simulink model "MX_Gateway"  (Simulink 9.0)
settings: Solver=FixedStepDiscrete  StartTime=0.0  StopTime=100  FixedStep=0.01  RelTol=1e-3
values from neighbouring files (symbols this model uses):
  ACTIVE = Bobcat.Parameter; CreateDefine( ACTIVE                         ,1                          ,'Define' ,'defines.h' )    [defines.m]
  ACTIVE_MODE_ONE = Bobcat.Parameter; CreateDefine( ACTIVE_MODE_ONE                ,19                         ,'Define' ,'defines.h' )    [defines.m]
  AUTHENTICATED = Bobcat.Parameter; CreateDefine( AUTHENTICATED                  ,1                          ,'Define' ,'defines.h' )    [defines.m]
  AUX_EMEA_CONTROLS = Bobcat.Parameter; CreateDefine( AUX_EMEA_CONTROLS  ,1   ,'Define' ,'defines.h' )    [enums.m]
  AUX_FLOW_HIGH = Bobcat.Parameter; CreateDefine( AUX_FLOW_HIGH           ,4  ,'Define' ,'defines.h' )    [enums.m]
  AUX_FLOW_INACTIVE = Bobcat.Parameter; CreateDefine( AUX_FLOW_INACTIVE       ,0  ,'Define' ,'defines.h' )    [enums.m]
  AUX_FLOW_LOW = Bobcat.Parameter; CreateDefine( AUX_FLOW_LOW            ,1  ,'Define' ,'defines.h' )    [enums.m]
  AUX_FLOW_MEDIUM = Bobcat.Parameter; CreateDefine( AUX_FLOW_MEDIUM         ,2  ,'Define' ,'defines.h' )    [enums.m]
  AUX_FLOW_STANDARD = Bobcat.Parameter; CreateDefine( AUX_FLOW_STANDARD       ,3  ,'Define' ,'defines.h' )    [enums.m]
  AUX_NON_FUNCTIONAL = Bobcat.Parameter; CreateDefine( AUX_NON_FUNCTIONAL ,255 ,'Define' ,'defines.h' )    [enums.m]
  BASE_ACTIVE = Bobcat.Parameter; CreateDefine( BASE_ACTIVE                    ,253                        ,'Define' ,'defines.h' )    [defines.m]
  BASE_DETENT = Bobcat.Parameter; CreateDefine( BASE_DETENT                    ,1                          ,'Define' ,'defines.h' )    [defines.m]
  BEEP_NONE = Bobcat.Parameter; CreateDefine( BEEP_NONE                      ,255                        ,'Define' ,'defines.h' )    [defines.m]
  CD_AIR_FILTER_DEBOUNCE_NORMAL = Bobcat.Parameter; CreateAliasCD( CD_AIR_FILTER_DEBOUNCE_NORMAL                        ,uint16(500)                ,'ConstantDataPointer' ,'CONSTANT_DATA' ,'AIR_FILTER_DEBOUNCE_NORMAL')    [cdata.m]    (also in hc12_cdata.m; m4_cdata.m)
  CD_AIR_FILTER_DEBOUNCE_NOT_CONNECTED = Bobcat.Parameter; CreateAliasCD( CD_AIR_FILTER_DEBOUNCE_NOT_CONNECTED                 ,uint16(3000)               ,'ConstantDataPointer' ,'CONSTANT_DATA' ,'AIR_FILTER_DEBOUNCE_NOT_CONNECTED')    [cdata.m]    (also in hc12_cdata.m; m4_cdata.m)
  CD_AIR_FILTER_DEBOUNCE_PLUGGED = Bobcat.Parameter; CreateAliasCD( CD_AIR_FILTER_DEBOUNCE_PLUGGED                       ,uint16(3000)               ,'ConstantDataPointer' ,'CONSTANT_DATA' ,'AIR_FILTER_DEBOUNCE_PLUGGED')    [cdata.m]    (also in hc12_cdata.m; m4_cdata.m)
  CD_AIR_FILTER_FUNCTION = Bobcat.Parameter; CreateAliasCD( CD_AIR_FILTER_FUNCTION                               ,uint8(255)                 ,'ConstantDataPointer' ,'CONSTANT_DATA' ,'AIR_FILTER_FUNCTION')    [cdata.m]    (also in hc12_cdata.m; m4_cdata.m)
  CD_AIR_FILTER_PLUGGED_WEIGHT = Bobcat.Parameter; CreateAliasCD( CD_AIR_FILTER_PLUGGED_WEIGHT                         ,uint8(2)                   ,'ConstantDataPointer' ,'CONSTANT_DATA' ,'AIR_FILTER_PLUGGED_WEIGHT')    [cdata.m]    (also in hc12_cdata.m; m4_cdata.m)
  CD_AIR_FILTER_UNPLUGGED_WEIGHT = Bobcat.Parameter; CreateAliasCD( CD_AIR_FILTER_UNPLUGGED_WEIGHT                       ,uint8(1)                   ,'ConstantDataPointer' ,'CONSTANT_DATA' ,'AIR_FILTER_UNPLUGGED_WEIGHT')    [cdata.m]    (also in hc12_cdata.m; m4_cdata.m)
  CD_AUTOIDLE_ARMBAR_DELAY = Bobcat.Parameter; CreateAliasCD( CD_AUTOIDLE_ARMBAR_DELAY                             ,uint8(2)                   ,'ConstantDataPointer' ,'CONSTANT_DATA' ,'AUTOIDLE_ARMBAR_DELAY')    [cdata.m]    (also in hc12_cdata.m; m4_cdata.m)
  CD_AUTOIDLE_FUNCTION = Bobcat.Parameter; CreateAliasCD( CD_AUTOIDLE_FUNCTION                                 ,uint8(255)                 ,'ConstantDataPointer' ,'CONSTANT_DATA' ,'AUTOIDLE_FUNCTION')    [cdata.m]    (also in hc12_cdata.m; m4_cdata.m)
  CD_AUTOIDLE_IDLE_DELAY = Bobcat.Parameter; CreateAliasCD( CD_AUTOIDLE_IDLE_DELAY                               ,uint8(40)                  ,'ConstantDataPointer' ,'CONSTANT_DATA' ,'AUTOIDLE_IDLE_DELAY')    [cdata.m]    (also in hc12_cdata.m; m4_cdata.m)
  CD_AUTOIDLE_LOW_IDLE_START = Bobcat.Parameter; CreateAliasCD( CD_AUTOIDLE_LOW_IDLE_START                           ,uint8(255)                 ,'ConstantDataPointer' ,'CONSTANT_DATA' ,'AUTOIDLE_LOW_IDLE_START')    [cdata.m]    (also in hc12_cdata.m; m4_cdata.m)
  CD_AUTOIDLE_PRESSURE_OFF = Bobcat.Parameter; CreateAliasCD( CD_AUTOIDLE_PRESSURE_OFF                             ,uint16(300)                ,'ConstantDataPointer' ,'CONSTANT_DATA' ,'AUTOIDLE_PRESSURE_OFF')    [cdata.m]    (also in hc12_cdata.m; m4_cdata.m)
  CD_AUTOIDLE_PRESSURE_ON = Bobcat.Parameter; CreateAliasCD( CD_AUTOIDLE_PRESSURE_ON                              ,uint16(270)                ,'ConstantDataPointer' ,'CONSTANT_DATA' ,'AUTOIDLE_PRESSURE_ON')    [cdata.m]    (also in hc12_cdata.m; m4_cdata.m)
  CD_AUTOSHIFT_FUNCTION = Bobcat.Parameter; CreateAliasCD( CD_AUTOSHIFT_FUNCTION                                ,uint8(255)                 ,'ConstantDataPointer' ,'CONSTANT_DATA' ,'AUTOSHIFT_FUNCTION')    [cdata.m]    (also in hc12_cdata.m; m4_cdata.m)
  CD_AUTOSHIFT_PHIGH1 = Bobcat.Parameter; CreateAliasCD( CD_AUTOSHIFT_PHIGH1                                  ,uint16(205)                ,'ConstantDataPointer' ,'CONSTANT_DATA' ,'AUTOSHIFT_PHIGH1')    [cdata.m]    (also in hc12_cdata.m; m4_cdata.m)
  CD_AUTOSHIFT_PHIGH2 = Bobcat.Parameter; CreateAliasCD( CD_AUTOSHIFT_PHIGH2                                  ,uint16(193)                ,'ConstantDataPointer' ,'CONSTANT_DATA' ,'AUTOSHIFT_PHIGH2')    [cdata.m]    (also in hc12_cdata.m; m4_cdata.m)
  CD_AUTOSHIFT_PHIGH_TEMP1 = Bobcat.Parameter; CreateAliasCD( CD_AUTOSHIFT_PHIGH_TEMP1                             ,int16(70)                  ,'ConstantDataPointer' ,'CONSTANT_DATA' ,'AUTOSHIFT_PHIGH_TEMP1')    [cdata.m]    (also in hc12_cdata.m; m4_cdata.m)
  CD_AUTOSHIFT_PHIGH_TEMP2 = Bobcat.Parameter; CreateAliasCD( CD_AUTOSHIFT_PHIGH_TEMP2                             ,int16(160)                 ,'ConstantDataPointer' ,'CONSTANT_DATA' ,'AUTOSHIFT_PHIGH_TEMP2')    [cdata.m]    (also in hc12_cdata.m; m4_cdata.m)
  CD_AUTOSHIFT_PLOW1 = Bobcat.Parameter; CreateAliasCD( CD_AUTOSHIFT_PLOW1                                   ,uint16(130)                ,'ConstantDataPointer' ,'CONSTANT_DATA' ,'AUTOSHIFT_PLOW1')    [cdata.m]    (also in hc12_cdata.m; m4_cdata.m)
  CD_AUTOSHIFT_PLOW2 = Bobcat.Parameter; CreateAliasCD( CD_AUTOSHIFT_PLOW2                                   ,uint16(100)                ,'ConstantDataPointer' ,'CONSTANT_DATA' ,'AUTOSHIFT_PLOW2')    [cdata.m]    (also in hc12_cdata.m; m4_cdata.m)
  CD_AUTOSHIFT_PLOW_TEMP1 = Bobcat.Parameter; CreateAliasCD( CD_AUTOSHIFT_PLOW_TEMP1                              ,int16(20)                  ,'ConstantDataPointer' ,'CONSTANT_DATA' ,'AUTOSHIFT_PLOW_TEMP1')    [cdata.m]    (also in hc12_cdata.m; m4_cdata.m)
  CD_AUTOSHIFT_PLOW_TEMP2 = Bobcat.Parameter; CreateAliasCD( CD_AUTOSHIFT_PLOW_TEMP2                              ,int16(80)                  ,'ConstantDataPointer' ,'CONSTANT_DATA' ,'AUTOSHIFT_PLOW_TEMP2')    [cdata.m]    (also in hc12_cdata.m; m4_cdata.m)
  CD_AUTOSHIFT_TRAVEL_DELAY = Bobcat.Parameter; CreateAliasCD( CD_AUTOSHIFT_TRAVEL_DELAY                            ,uint8(32)                  ,'ConstantDataPointer' ,'CONSTANT_DATA' ,'AUTOSHIFT_TRAVEL_DELAY')    [cdata.m]    (also in hc12_cdata.m; m4_cdata.m)
  CD_AUX2_CRACKPOINT_CURRENT = Bobcat.Parameter; CreateAliasCD( CD_AUX2_CRACKPOINT_CURRENT                           ,fi(0,0,16,12,'hex','0c28') ,'ConstantDataPointer' ,'CONSTANT_DATA' ,'AUX2_CRACKPOINT_CURRENT')    [cdata.m]    (also in hc12_cdata.m; m4_cdata.m)
  CD_AUX2_FLOW_LOW_FUNCTION = Bobcat.Parameter; CreateAliasCD( CD_AUX2_FLOW_LOW_FUNCTION                            ,uint8(255)                 ,'ConstantDataPointer' ,'CONSTANT_DATA' ,'AUX2_FLOW_LOW_FUNCTION')    [cdata.m]    (also in hc12_cdata.m; m4_cdata.m)
  CD_AUX2_FLOW_MED_FUNCTION = Bobcat.Parameter; CreateAliasCD( CD_AUX2_FLOW_MED_FUNCTION                            ,uint8(255)                 ,'ConstantDataPointer' ,'CONSTANT_DATA' ,'AUX2_FLOW_MED_FUNCTION')    [cdata.m]    (also in hc12_cdata.m; m4_cdata.m)
  CD_AUX2_HI_FLOW_PERCENT = Bobcat.Parameter; CreateAliasCD( CD_AUX2_HI_FLOW_PERCENT                              ,fi(0,0,16,16,'hex','ffff') ,'ConstantDataPointer' ,'CONSTANT_DATA' ,'AUX2_HI_FLOW_PERCENT')    [cdata.m]    (also in hc12_cdata.m; m4_cdata.m)
  CD_AUX2_LOW_FLOW_PERCENT = Bobcat.Parameter; CreateAliasCD( CD_AUX2_LOW_FLOW_PERCENT                             ,fi(0,0,16,16,'hex','7333') ,'ConstantDataPointer' ,'CONSTANT_DATA' ,'AUX2_LOW_FLOW_PERCENT')    [cdata.m]    (also in hc12_cdata.m; m4_cdata.m)
  CD_AUX2_MAX_FLOW_CURRENT = Bobcat.Parameter; CreateAliasCD( CD_AUX2_MAX_FLOW_CURRENT                             ,fi(0,0,16,12,'hex','14cc') ,'ConstantDataPointer' ,'CONSTANT_DATA' ,'AUX2_MAX_FLOW_CURRENT')    [cdata.m]    (also in hc12_cdata.m; m4_cdata.m)
  CD_AUX2_MED_FLOW_PERCENT = Bobcat.Parameter; CreateAliasCD( CD_AUX2_MED_FLOW_PERCENT                             ,fi(0,0,16,16,'hex','9999') ,'ConstantDataPointer' ,'CONSTANT_DATA' ,'AUX2_MED_FLOW_PERCENT')    [cdata.m]    (also in hc12_cdata.m; m4_cdata.m)
  CD_AUX_CRACKPOINT_CURRENT = Bobcat.Parameter; CreateAliasCD( CD_AUX_CRACKPOINT_CURRENT                            ,fi(0,0,16,12,'hex','0000') ,'ConstantDataPointer' ,'CONSTANT_DATA' ,'AUX_CRACKPOINT_CURRENT')    [cdata.m]    (also in hc12_cdata.m; m4_cdata.m)
  CD_AUX_DETENT_FLASH_TIME = Bobcat.Parameter; CreateAliasCD( CD_AUX_DETENT_FLASH_TIME                             ,uint16(72)                 ,'ConstantDataPointer' ,'CONSTANT_DATA' ,'AUX_DETENT_FLASH_TIME')    [cdata.m]    (also in hc12_cdata.m; m4_cdata.m)
  CD_AUX_DETENT_HOLD_TIME = Bobcat.Parameter; CreateAliasCD( CD_AUX_DETENT_HOLD_TIME                              ,uint16(100)                ,'ConstantDataPointer' ,'CONSTANT_DATA' ,'AUX_DETENT_HOLD_TIME')    [cdata.m]    (also in hc12_cdata.m; m4_cdata.m)
  CD_AUX_FULL_ON_AUX1 = Bobcat.Parameter; CreateAliasCD( CD_AUX_FULL_ON_AUX1                                  ,fi(0,0,16,12,'hex','10cd') ,'ConstantDataPointer' ,'CONSTANT_DATA' ,'AUX_FULL_ON_AUX1')    [cdata.m]    (also in hc12_cdata.m; m4_cdata.m)
  CD_AUX_FULL_ON_AUX2 = Bobcat.Parameter; CreateAliasCD( CD_AUX_FULL_ON_AUX2                                  ,fi(0,0,16,12,'hex','139d') ,'ConstantDataPointer' ,'CONSTANT_DATA' ,'AUX_FULL_ON_AUX2')    [cdata.m]    (also in hc12_cdata.m; m4_cdata.m)
  CD_AUX_FULL_ON_AUX3 = Bobcat.Parameter; CreateAliasCD( CD_AUX_FULL_ON_AUX3                                  ,fi(0,0,16,12,'hex','17d7') ,'ConstantDataPointer' ,'CONSTANT_DATA' ,'AUX_FULL_ON_AUX3')    [cdata.m]    (also in hc12_cdata.m; m4_cdata.m)
  CD_AUX_FUNCTION = Bobcat.Parameter; CreateAliasCD( CD_AUX_FUNCTION                                      ,uint8(0)                   ,'ConstantDataPointer' ,'CONSTANT_DATA' ,'AUX_FUNCTION')    [cdata.m]    (also in hc12_cdata.m; m4_cdata.m)
  CD_AUX_RATE_LIMIT_CRACKPOINT = Bobcat.Parameter; CreateAliasCD( CD_AUX_RATE_LIMIT_CRACKPOINT                         ,fi(0,0,16,12,'hex','0800') ,'ConstantDataPointer' ,'CONSTANT_DATA' ,'AUX_RATE_LIMIT_CRACKPOINT')    [cdata.m]    (also in hc12_cdata.m; m4_cdata.m)
  CD_AUX_RATE_LIMIT_CRACKPOINT_RATE = Bobcat.Parameter; CreateAliasCD( CD_AUX_RATE_LIMIT_CRACKPOINT_RATE                    ,fi(0,1,32,12,'int',102400) ,'ConstantDataPointer' ,'CONSTANT_DATA' ,'AUX_RATE_LIMIT_CRACKPOINT_RATE')    [cdata.m]    (also in hc12_cdata.m; m4_cdata.m)
  CD_AUX_RATE_LIMIT_FUNCTION = Bobcat.Parameter; CreateAliasCD( CD_AUX_RATE_LIMIT_FUNCTION                           ,uint8(255)                 ,'ConstantDataPointer' ,'CONSTANT_DATA' ,'AUX_RATE_LIMIT_FUNCTION')    [cdata.m]    (also in hc12_cdata.m; m4_cdata.m)
  CD_AUX_RATE_LIMIT_MAX_ENG_SPD = Bobcat.Parameter; CreateAliasCD( CD_AUX_RATE_LIMIT_MAX_ENG_SPD                        ,uint16(1600)               ,'ConstantDataPointer' ,'CONSTANT_DATA' ,'AUX_RATE_LIMIT_MAX_ENG_SPD')    [cdata.m]    (also in hc12_cdata.m; m4_cdata.m)
  CD_AUX_RATE_LIMIT_MIN_ENG_SPD = Bobcat.Parameter; CreateAliasCD( CD_AUX_RATE_LIMIT_MIN_ENG_SPD                        ,uint16(100)                ,'ConstantDataPointer' ,'CONSTANT_DATA' ,'AUX_RATE_LIMIT_MIN_ENG_SPD')    [cdata.m]    (also in hc12_cdata.m; m4_cdata.m)
  CD_AUX_RATE_LIMIT_NORMAL_RATE = Bobcat.Parameter; CreateAliasCD( CD_AUX_RATE_LIMIT_NORMAL_RATE                        ,fi(0,1,32,12,'int',2048)   ,'ConstantDataPointer' ,'CONSTANT_DATA' ,'AUX_RATE_LIMIT_NORMAL_RATE')    [cdata.m]    (also in hc12_cdata.m; m4_cdata.m)
  CD_AUXPOT_CAL_DEADBAND_HIGH = Bobcat.Parameter; CreateAliasCD( CD_AUXPOT_CAL_DEADBAND_HIGH                          ,uint16(574)                ,'ConstantDataPointer' ,'CONSTANT_DATA' ,'AUXPOT_CAL_DEADBAND_HIGH')    [cdata.m]    (also in hc12_cdata.m; m4_cdata.m)
  CD_AUXPOT_CAL_DEADBAND_LOW = Bobcat.Parameter; CreateAliasCD( CD_AUXPOT_CAL_DEADBAND_LOW                           ,uint16(424)                ,'ConstantDataPointer' ,'CONSTANT_DATA' ,'AUXPOT_CAL_DEADBAND_LOW')    [cdata.m]    (also in hc12_cdata.m; m4_cdata.m)
  CD_AUXPOT_CENTER_RATIO = Bobcat.Parameter; CreateAliasCD( CD_AUXPOT_CENTER_RATIO                               ,uint16(500)                ,'ConstantDataPointer' ,'CONSTANT_DATA' ,'AUXPOT_CENTER_RATIO')    [cdata.m]    (also in hc12_cdata.m; m4_cdata.m)
  CD_AUXPOT_DEADBAND = Bobcat.Parameter; CreateAliasCD( CD_AUXPOT_DEADBAND                                   ,uint8(38)                  ,'ConstantDataPointer' ,'CONSTANT_DATA' ,'AUXPOT_DEADBAND')    [cdata.m]    (also in hc12_cdata.m; m4_cdata.m)
  CD_AUXPOT_FUNCTION = Bobcat.Parameter; CreateAliasCD( CD_AUXPOT_FUNCTION                                   ,uint8(0)                   ,'ConstantDataPointer' ,'CONSTANT_DATA' ,'AUXPOT_FUNCTION')    [cdata.m]    (also in hc12_cdata.m; m4_cdata.m)
  CD_AUXPOT_RATIO_GAIN = Bobcat.Parameter; CreateAliasCD( CD_AUXPOT_RATIO_GAIN                                 ,uint16(1000)               ,'ConstantDataPointer' ,'CONSTANT_DATA' ,'AUXPOT_RATIO_GAIN')    [cdata.m]    (also in hc12_cdata.m; m4_cdata.m)
  CD_CAN_THROTTLE_FUNCTION = Bobcat.Parameter; CreateAliasCD( CD_CAN_THROTTLE_FUNCTION                             ,uint8(255)                 ,'ConstantDataPointer' ,'CONSTANT_DATA' ,'CAN_THROTTLE_FUNCTION')    [cdata.m]    (also in hc12_cdata.m; m4_cdata.m)
  CD_CAN_THROTTLE_REVERSE = Bobcat.Parameter; CreateAliasCD( CD_CAN_THROTTLE_REVERSE                              ,uint8(255)                 ,'ConstantDataPointer' ,'CONSTANT_DATA' ,'CAN_THROTTLE_REVERSE')    [cdata.m]    (also in hc12_cdata.m; m4_cdata.m)
  CD_CONSOLE_DEBOUNCE_ABSENT = Bobcat.Parameter; CreateAliasCD( CD_CONSOLE_DEBOUNCE_ABSENT                           ,uint16(12)                 ,'ConstantDataPointer' ,'CONSTANT_DATA' ,'CONSOLE_DEBOUNCE_ABSENT')    [cdata.m]    (also in hc12_cdata.m; m4_cdata.m)
  CD_CONSOLE_DEBOUNCE_OORH = Bobcat.Parameter; CreateAliasCD( CD_CONSOLE_DEBOUNCE_OORH                             ,uint16(12)                 ,'ConstantDataPointer' ,'CONSTANT_DATA' ,'CONSOLE_DEBOUNCE_OORH')    [cdata.m]    (also in hc12_cdata.m; m4_cdata.m)
  CD_CONSOLE_DEBOUNCE_OORL = Bobcat.Parameter; CreateAliasCD( CD_CONSOLE_DEBOUNCE_OORL                             ,uint16(12)                 ,'ConstantDataPointer' ,'CONSTANT_DATA' ,'CONSOLE_DEBOUNCE_OORL')    [cdata.m]    (also in hc12_cdata.m; m4_cdata.m)
  CD_CONSOLE_DEBOUNCE_PRESENT = Bobcat.Parameter; CreateAliasCD( CD_CONSOLE_DEBOUNCE_PRESENT                          ,uint16(12)                 ,'ConstantDataPointer' ,'CONSTANT_DATA' ,'CONSOLE_DEBOUNCE_PRESENT')    [cdata.m]    (also in hc12_cdata.m; m4_cdata.m)
  CD_CONSOLE_FUNCTION = Bobcat.Parameter; CreateAliasCD( CD_CONSOLE_FUNCTION                                  ,uint8(0)                   ,'ConstantDataPointer' ,'CONSTANT_DATA' ,'CONSOLE_FUNCTION')    [cdata.m]    (also in hc12_cdata.m; m4_cdata.m)
  CD_CONSOLE_OORH = Bobcat.Parameter; CreateAliasCD( CD_CONSOLE_OORH                                      ,uint16(740)                ,'ConstantDataPointer' ,'CONSTANT_DATA' ,'CONSOLE_OORH')    [cdata.m]    (also in hc12_cdata.m; m4_cdata.m)
  CD_CONSOLE_OORL = Bobcat.Parameter; CreateAliasCD( CD_CONSOLE_OORL                                      ,uint16(10)                 ,'ConstantDataPointer' ,'CONSTANT_DATA' ,'CONSOLE_OORL')    [cdata.m]    (also in hc12_cdata.m; m4_cdata.m)
  CD_CONSOLE_PRESENT_HIGH = Bobcat.Parameter; CreateAliasCD( CD_CONSOLE_PRESENT_HIGH                              ,uint16(515)                ,'ConstantDataPointer' ,'CONSTANT_DATA' ,'CONSOLE_PRESENT_HIGH')    [cdata.m]    (also in hc12_cdata.m; m4_cdata.m)
  CD_CONSOLE_PRESENT_LOW = Bobcat.Parameter; CreateAliasCD( CD_CONSOLE_PRESENT_LOW                               ,uint16(375)                ,'ConstantDataPointer' ,'CONSTANT_DATA' ,'CONSOLE_PRESENT_LOW')    [cdata.m]    (also in hc12_cdata.m; m4_cdata.m)
  CD_DELUXE_GEN5_5_FUNCTION = Bobcat.Parameter; CreateAliasCD( CD_DELUXE_GEN5_5_FUNCTION                            ,uint8(255)                 ,'ConstantDataPointer' ,'CONSTANT_DATA' ,'DELUXE_GEN5_5_FUNCTION')    [cdata.m]    (also in hc12_cdata.m; m4_cdata.m)
  CD_DELUXE_GEN5_FUNCTION = Bobcat.Parameter; CreateAliasCD( CD_DELUXE_GEN5_FUNCTION                              ,uint8(255)                 ,'ConstantDataPointer' ,'CONSTANT_DATA' ,'DELUXE_GEN5_FUNCTION')    [cdata.m]    (also in hc12_cdata.m; m4_cdata.m)
  CD_DERATE_ENGINE_SPEED_DEBOUNCE_HIGH = Bobcat.Parameter; CreateAliasCD( CD_DERATE_ENGINE_SPEED_DEBOUNCE_HIGH                 ,uint16(200)                ,'ConstantDataPointer' ,'CONSTANT_DATA' ,'DERATE_ENGINE_SPEED_DEBOUNCE_HIGH')    [cdata.m]    (also in hc12_cdata.m; m4_cdata.m)
  CD_DERATE_ENGINE_SPEED_DEBOUNCE_SHUTDOWN = Bobcat.Parameter; CreateAliasCD( CD_DERATE_ENGINE_SPEED_DEBOUNCE_SHUTDOWN             ,uint16(1000)               ,'ConstantDataPointer' ,'CONSTANT_DATA' ,'DERATE_ENGINE_SPEED_DEBOUNCE_SHUTDOWN')    [cdata.m]    (also in hc12_cdata.m; m4_cdata.m)
  CD_DERATE_ENGINE_SPEED_HIGH_OFFSET = Bobcat.Parameter; CreateAliasCD( CD_DERATE_ENGINE_SPEED_HIGH_OFFSET                   ,uint16(200)                ,'ConstantDataPointer' ,'CONSTANT_DATA' ,'DERATE_ENGINE_SPEED_HIGH_OFFSET')    [cdata.m]    (also in hc12_cdata.m; m4_cdata.m)
  CD_DERATE_METHOD = Bobcat.Parameter; CreateAliasCD( CD_DERATE_METHOD                                     ,uint8(0)                   ,'ConstantDataPointer' ,'CONSTANT_DATA' ,'DERATE_METHOD')    [cdata.m]    (also in hc12_cdata.m; m4_cdata.m)
  CD_DUMP_VALVE_FUNCTION = Bobcat.Parameter; CreateAliasCD( CD_DUMP_VALVE_FUNCTION                               ,uint8(255)                 ,'ConstantDataPointer' ,'CONSTANT_DATA' ,'DUMP_VALVE_FUNCTION')    [cdata.m]    (also in hc12_cdata.m; m4_cdata.m)
  CD_DUMP_VALVE_ON_CURRENT = Bobcat.Parameter; CreateAliasCD( CD_DUMP_VALVE_ON_CURRENT                             ,fi(0,0,16,12,'hex','ffff') ,'ConstantDataPointer' ,'CONSTANT_DATA' ,'DUMP_VALVE_ON_CURRENT')    [cdata.m]    (also in hc12_cdata.m; m4_cdata.m)
  CD_ECO_MODE_THROTTLE_REDUCTION = Bobcat.Parameter; CreateAliasCD( CD_ECO_MODE_THROTTLE_REDUCTION                       ,uint16(850)                ,'ConstantDataPointer' ,'CONSTANT_DATA' ,'ECO_MODE_THROTTLE_REDUCTION')    [cdata.m]    (also in hc12_cdata.m; m4_cdata.m)
  CD_ECT_FILTER_GAIN = Bobcat.Parameter; CreateAliasCD( CD_ECT_FILTER_GAIN                                   ,uint8(6)                   ,'ConstantDataPointer' ,'CONSTANT_DATA' ,'ECT_FILTER_GAIN')    [cdata.m]    (also in hc12_cdata.m; m4_cdata.m)
  CD_ECU_FUNCTION = Bobcat.Parameter; CreateAliasCD( CD_ECU_FUNCTION                                      ,uint8(255)                 ,'ConstantDataPointer' ,'CONSTANT_DATA' ,'ECU_FUNCTION')    [cdata.m]    (also in hc12_cdata.m; m4_cdata.m)
  CD_ECU_HOLD_DEBOUNCE_ERROR_ON = Bobcat.Parameter; CreateAliasCD( CD_ECU_HOLD_DEBOUNCE_ERROR_ON                        ,uint16(20)                 ,'ConstantDataPointer' ,'CONSTANT_DATA' ,'ECU_HOLD_DEBOUNCE_ERROR_ON')    [cdata.m]    (also in hc12_cdata.m; m4_cdata.m)
  CD_EMEA_CONTROLS_HOLD_TIME = Bobcat.Parameter; CreateAliasCD( CD_EMEA_CONTROLS_HOLD_TIME                           ,uint16(30)                 ,'ConstantDataPointer' ,'CONSTANT_DATA' ,'EMEA_CONTROLS_HOLD_TIME')    [cdata.m]    (also in hc12_cdata.m; m4_cdata.m)
  CD_EMEA_CONTROLS_OPTION_A = Bobcat.Parameter; CreateAliasCD( CD_EMEA_CONTROLS_OPTION_A                            ,uint8(255)                 ,'ConstantDataPointer' ,'CONSTANT_DATA' ,'EMEA_CONTROLS_OPTION_A')    [cdata.m]    (also in hc12_cdata.m; m4_cdata.m)
  CD_EMEA_CONTROLS_OPTION_C = Bobcat.Parameter; CreateAliasCD( CD_EMEA_CONTROLS_OPTION_C                            ,uint8(255)                 ,'ConstantDataPointer' ,'CONSTANT_DATA' ,'EMEA_CONTROLS_OPTION_C')    [cdata.m]    (also in hc12_cdata.m; m4_cdata.m)
  CD_EMEA_CONTROLS_REVERSE = Bobcat.Parameter; CreateAliasCD( CD_EMEA_CONTROLS_REVERSE                             ,uint8(255)                 ,'ConstantDataPointer' ,'CONSTANT_DATA' ,'EMEA_CONTROLS_REVERSE')    [cdata.m]    (also in hc12_cdata.m; m4_cdata.m)
  CD_EMEA_ELECTRIC_VERSION = Bobcat.Parameter; CreateAliasCD( CD_EMEA_ELECTRIC_VERSION                             ,uint8(0)                   ,'ConstantDataPointer' ,'CONSTANT_DATA' ,'EMEA_ELECTRIC_VERSION')    [cdata.m]    (also in hc12_cdata.m; m4_cdata.m)
  CD_EMEA_POT_CAL_DEADBAND_HIGH = Bobcat.Parameter; CreateAliasCD( CD_EMEA_POT_CAL_DEADBAND_HIGH                        ,uint16(574)                ,'ConstantDataPointer' ,'CONSTANT_DATA' ,'EMEA_POT_CAL_DEADBAND_HIGH')    [cdata.m]    (also in hc12_cdata.m; m4_cdata.m)
  CD_EMEA_POT_CAL_DEADBAND_LOW = Bobcat.Parameter; CreateAliasCD( CD_EMEA_POT_CAL_DEADBAND_LOW                         ,uint16(424)                ,'ConstantDataPointer' ,'CONSTANT_DATA' ,'EMEA_POT_CAL_DEADBAND_LOW')    [cdata.m]    (also in hc12_cdata.m; m4_cdata.m)
  CD_ENGINE_RPM_FACTOR = Bobcat.Parameter; CreateAliasCD( CD_ENGINE_RPM_FACTOR                                 ,uint8(35)                  ,'ConstantDataPointer' ,'CONSTANT_DATA' ,'ENGINE_RPM_FACTOR')    [cdata.m]    (also in hc12_cdata.m; m4_cdata.m)
  CD_ENGINE_SPEED_FILTER_FAST_VALUE = Bobcat.Parameter; CreateAliasCD( CD_ENGINE_SPEED_FILTER_FAST_VALUE                    ,uint8(1)                   ,'ConstantDataPointer' ,'CONSTANT_DATA' ,'ENGINE_SPEED_FILTER_FAST_VALUE')    [cdata.m]    (also in hc12_cdata.m; m4_cdata.m)
  CD_ENGINE_SPEED_FILTER_SLOW_VALUE = Bobcat.Parameter; CreateAliasCD( CD_ENGINE_SPEED_FILTER_SLOW_VALUE                    ,uint8(4)                   ,'ConstantDataPointer' ,'CONSTANT_DATA' ,'ENGINE_SPEED_FILTER_SLOW_VALUE')    [cdata.m]    (also in hc12_cdata.m; m4_cdata.m)
  CD_ENGINE_SPEED_RUNNING = Bobcat.Parameter; CreateAliasCD( CD_ENGINE_SPEED_RUNNING                              ,uint16(800)                ,'ConstantDataPointer' ,'CONSTANT_DATA' ,'ENGINE_SPEED_RUNNING')    [cdata.m]    (also in hc12_cdata.m; m4_cdata.m)
  CD_ENGINE_START_DELAY_DEBOUNCE_RPM_NO_SIGNAL = Bobcat.Parameter; CreateAliasCD( CD_ENGINE_START_DELAY_DEBOUNCE_RPM_NO_SIGNAL         ,uint16(0)                  ,'ConstantDataPointer' ,'CONSTANT_DATA' ,'ENGINE_START_DELAY_DEBOUNCE_RPM_NO_SIGNAL')    [cdata.m]    (also in hc12_cdata.m; m4_cdata.m)
  CD_ENGINE_START_DELAY_OFF_TIME = Bobcat.Parameter; CreateAliasCD( CD_ENGINE_START_DELAY_OFF_TIME                       ,uint16(0)                  ,'ConstantDataPointer' ,'CONSTANT_DATA' ,'ENGINE_START_DELAY_OFF_TIME')    [cdata.m]    (also in hc12_cdata.m; m4_cdata.m)
  CD_ENGINE_START_DELAY_RPM_NO_SIGNAL = Bobcat.Parameter; CreateAliasCD( CD_ENGINE_START_DELAY_RPM_NO_SIGNAL                  ,uint16(0)                  ,'ConstantDataPointer' ,'CONSTANT_DATA' ,'ENGINE_START_DELAY_RPM_NO_SIGNAL')    [cdata.m]    (also in hc12_cdata.m; m4_cdata.m)
  CD_EOP_DEBOUNCE_EXTREMELY_LOW = Bobcat.Parameter; CreateAliasCD( CD_EOP_DEBOUNCE_EXTREMELY_LOW                        ,uint16(50)                 ,'ConstantDataPointer' ,'CONSTANT_DATA' ,'EOP_DEBOUNCE_EXTREMELY_LOW')    [cdata.m]    (also in hc12_cdata.m; m4_cdata.m)
  CD_EOP_DEBOUNCE_NORMAL = Bobcat.Parameter; CreateAliasCD( CD_EOP_DEBOUNCE_NORMAL                               ,uint16(50)                 ,'ConstantDataPointer' ,'CONSTANT_DATA' ,'EOP_DEBOUNCE_NORMAL')    [cdata.m]    (also in hc12_cdata.m; m4_cdata.m)
  CD_EOP_DEBOUNCE_SHUTDOWN = Bobcat.Parameter; CreateAliasCD( CD_EOP_DEBOUNCE_SHUTDOWN                             ,uint16(100)                ,'ConstantDataPointer' ,'CONSTANT_DATA' ,'EOP_DEBOUNCE_SHUTDOWN')    [cdata.m]    (also in hc12_cdata.m; m4_cdata.m)
  CD_EOP_FILTER_VALUE = Bobcat.Parameter; CreateAliasCD( CD_EOP_FILTER_VALUE                                  ,uint8(3)                   ,'ConstantDataPointer' ,'CONSTANT_DATA' ,'EOP_FILTER_VALUE')    [cdata.m]    (also in hc12_cdata.m; m4_cdata.m)
  CD_EOP_FUNCTION = Bobcat.Parameter; CreateAliasCD( CD_EOP_FUNCTION                                      ,uint8(1)                   ,'ConstantDataPointer' ,'CONSTANT_DATA' ,'EOP_FUNCTION')    [cdata.m]    (also in hc12_cdata.m; m4_cdata.m)
  CD_EOP_SWITCH_GAUGE_VALUE = Bobcat.Parameter; CreateAliasCD( CD_EOP_SWITCH_GAUGE_VALUE                            ,uint16(125)                ,'ConstantDataPointer' ,'CONSTANT_DATA' ,'EOP_SWITCH_GAUGE_VALUE')    [cdata.m]    (also in hc12_cdata.m; m4_cdata.m)
  CD_EOP_SWITCH_LOW = Bobcat.Parameter; CreateAliasCD( CD_EOP_SWITCH_LOW                                    ,uint16(50)                 ,'ConstantDataPointer' ,'CONSTANT_DATA' ,'EOP_SWITCH_LOW')    [cdata.m]    (also in hc12_cdata.m; m4_cdata.m)
  CD_EOP_SWITCH_PRESSURE_VALUE = Bobcat.Parameter; CreateAliasCD( CD_EOP_SWITCH_PRESSURE_VALUE                         ,uint16(40)                 ,'ConstantDataPointer' ,'CONSTANT_DATA' ,'EOP_SWITCH_PRESSURE_VALUE')    [cdata.m]    (also in hc12_cdata.m; m4_cdata.m)
  CD_EOT_DEBOUNCE_EXTREMELY_LOW = Bobcat.Parameter; CreateAliasCD( CD_EOT_DEBOUNCE_EXTREMELY_LOW                        ,uint16(2)                  ,'ConstantDataPointer' ,'CONSTANT_DATA' ,'EOT_DEBOUNCE_EXTREMELY_LOW')    [cdata.m]    (also in hc12_cdata.m; m4_cdata.m)
  CD_EOT_DEBOUNCE_LOW = Bobcat.Parameter; CreateAliasCD( CD_EOT_DEBOUNCE_LOW                                  ,uint16(2)                  ,'ConstantDataPointer' ,'CONSTANT_DATA' ,'EOT_DEBOUNCE_LOW')    [cdata.m]    (also in hc12_cdata.m; m4_cdata.m)
  CD_EOT_DEBOUNCE_NORMAL = Bobcat.Parameter; CreateAliasCD( CD_EOT_DEBOUNCE_NORMAL                               ,uint16(2)                  ,'ConstantDataPointer' ,'CONSTANT_DATA' ,'EOT_DEBOUNCE_NORMAL')    [cdata.m]    (also in hc12_cdata.m; m4_cdata.m)
  CD_EOT_EXTREMELY_LOW = Bobcat.Parameter; CreateAliasCD( CD_EOT_EXTREMELY_LOW                                 ,uint16(81)                 ,'ConstantDataPointer' ,'CONSTANT_DATA' ,'EOT_EXTREMELY_LOW')    [cdata.m]    (also in hc12_cdata.m; m4_cdata.m)
  CD_EOT_FUNCTION = Bobcat.Parameter; CreateAliasCD( CD_EOT_FUNCTION                                      ,uint8(255)                 ,'ConstantDataPointer' ,'CONSTANT_DATA' ,'EOT_FUNCTION')    [cdata.m]    (also in hc12_cdata.m; m4_cdata.m)
  CD_EOT_LOW = Bobcat.Parameter; CreateAliasCD( CD_EOT_LOW                                           ,uint16(90)                 ,'ConstantDataPointer' ,'CONSTANT_DATA' ,'EOT_LOW')    [cdata.m]    (also in hc12_cdata.m; m4_cdata.m)
  CD_EOT_TEMPERATURE_HYSTERESIS = Bobcat.Parameter; CreateAliasCD( CD_EOT_TEMPERATURE_HYSTERESIS                        ,uint16(2)                  ,'ConstantDataPointer' ,'CONSTANT_DATA' ,'EOT_TEMPERATURE_HYSTERESIS')    [cdata.m]    (also in hc12_cdata.m; m4_cdata.m)
  CD_FAST_TRAVEL_FUNCTION = Bobcat.Parameter; CreateAliasCD( CD_FAST_TRAVEL_FUNCTION                              ,uint8(255)                 ,'ConstantDataPointer' ,'CONSTANT_DATA' ,'FAST_TRAVEL_FUNCTION')    [cdata.m]    (also in hc12_cdata.m; m4_cdata.m)
  CD_FAST_TRAVEL_THROTTLE_DELAY = Bobcat.Parameter; CreateAliasCD( CD_FAST_TRAVEL_THROTTLE_DELAY                        ,uint16(10)                 ,'ConstantDataPointer' ,'CONSTANT_DATA' ,'FAST_TRAVEL_THROTTLE_DELAY')    [cdata.m]    (also in hc12_cdata.m; m4_cdata.m)
  CD_FAST_TRAVEL_THROTTLE_LIMIT = Bobcat.Parameter; CreateAliasCD( CD_FAST_TRAVEL_THROTTLE_LIMIT                        ,uint16(1000)               ,'ConstantDataPointer' ,'CONSTANT_DATA' ,'FAST_TRAVEL_THROTTLE_LIMIT')    [cdata.m]    (also in hc12_cdata.m; m4_cdata.m)
  CD_FLYWHEEL_TEETH = Bobcat.Parameter; CreateAliasCD( CD_FLYWHEEL_TEETH                                    ,uint8(83)                  ,'ConstantDataPointer' ,'CONSTANT_DATA' ,'FLYWHEEL_TEETH')    [cdata.m]    (also in hc12_cdata.m; m4_cdata.m)
  CD_FUEL_LEVEL_FILTER_FAST_VALUE = Bobcat.Parameter; CreateAliasCD( CD_FUEL_LEVEL_FILTER_FAST_VALUE                      ,uint8(5)                   ,'ConstantDataPointer' ,'CONSTANT_DATA' ,'FUEL_LEVEL_FILTER_FAST_VALUE')    [cdata.m]    (also in hc12_cdata.m; m4_cdata.m)
  CD_FUEL_LEVEL_FILTER_SLOW_VALUE = Bobcat.Parameter; CreateAliasCD( CD_FUEL_LEVEL_FILTER_SLOW_VALUE                      ,uint8(10)                  ,'ConstantDataPointer' ,'CONSTANT_DATA' ,'FUEL_LEVEL_FILTER_SLOW_VALUE')    [cdata.m]    (also in hc12_cdata.m; m4_cdata.m)
  CD_FUEL_PRIMARY_FEEDBACK_FUNCTION = Bobcat.Parameter; CreateAliasCD( CD_FUEL_PRIMARY_FEEDBACK_FUNCTION                    ,uint8(0)                   ,'ConstantDataPointer' ,'CONSTANT_DATA' ,'FUEL_PRIMARY_FEEDBACK_FUNCTION')    [cdata.m]    (also in hc12_cdata.m; m4_cdata.m)
  ... (+246 more)
undefined symbols (not defined in the file or in its folder): sigbuilder_block
undefined symbols (not defined in the file): CD_FUEL_RESOLUTION_TIMER
libraries: simulink x186, MX_Library x107
(part 19 of 48)

== system MX_Gateway/MX_Gtwy_Control/System/Keyless_Entry ==
  note: Moved this into C-code since display wants to complicate things and require hash
  can_KeylessEntry : Inport
  state_KeylessEntryLock : Inport  Port=2
  state_KeylessEntryPassword : Inport  Port=3
  din_SwPowerRelay : Inport  Port=4
  din_RunEnter : Inport  Port=5
  state_Engine : Inport  Port=6
  Enable : EnablePort  StatesWhenEnabling=reset
  CabKeyDetection : Stateflow chart  TreatAsAtomicUnit=on
  Indicators : Stateflow chart  TreatAsAtomicUnit=on
  Password_Entry : Stateflow chart  Commented=on  TreatAsAtomicUnit=on
  Power_Hold : Stateflow chart  TreatAsAtomicUnit=on
  state_KeylessEntryPowerHold : Outport  InitialOutput=[0]
  ind_KeylessEntryLock : Outport  Port=2  OutputWhenDisabled=reset  InitialOutput=[0]
  ind_KeylessEntryUnlock : Outport  Port=3  OutputWhenDisabled=reset  InitialOutput=[0]
  cabKeyDetect : Outport  Port=4  InitialOutput=[0]
  wires:
  can_KeylessEntry/1 -> Power_Hold/1(can_KeylessEntry)
  Power_Hold/1(state_KeylessEntryPowerHold) -> state_KeylessEntryPowerHold/1
  Indicators/1(ind_KeylessEntryLock) -> ind_KeylessEntryLock/1
  Indicators/2(ind_KeylessEntryUnlock) -> ind_KeylessEntryUnlock/1
  state_KeylessEntryPassword/1 -> Indicators/1(state_KeylessEntryPassword), Power_Hold/3(state_KeylessEntryPassword)
  state_KeylessEntryLock/1 -> Indicators/2(state_KeylessEntryLock)
  CabKeyDetection/1(cabKeyDetect) -> [cabKeyDetect], cabKeyDetect/1
  state_Engine/1 -> CabKeyDetection/1(state_Engine)
  din_RunEnter/1 -> CabKeyDetection/2(din_RunEnter)
  din_SwPowerRelay/1 -> CabKeyDetection/3(din_SwPowerRelay)
  [cabKeyDetect] -> Power_Hold/2(cabKeyDetect)

== system MX_Gateway/MX_Gtwy_Control/System/Keyless_Start ==
  can_Keypad : Inport
  state_KeylessPanel : Inport  Port=2
  ee_MasterPassword : Inport  Port=3
  ee_OwnerPassword : Inport  Port=4
  ee_User1Password : Inport  Port=5
  ee_User2Password : Inport  Port=6
  flag_InitEERead : Inport  Port=7
  state_MachineLock : Inport  Port=8
  state_Engine : Inport  Port=9
  Panel_Lock : Stateflow chart  TreatAsAtomicUnit=on
  state_KSLock : Outport
  state_KSPassword : Outport  Port=2
  pwd_Position : Outport  Port=3
  pwd_Keyless : Outport  Port=4
  KSS_Display : Outport  Port=5
  PWD_NumberSelected : Outport  Port=6
  wires:
  ee_MasterPassword/1 -> Panel_Lock/4(ee_MasterPassword)  "ee_MasterPassword"
  ee_OwnerPassword/1 -> Panel_Lock/3(ee_OwnerPassword)  "ee_OwnerPasswrod"
  Panel_Lock/4(pwd_Keyless) -> pwd_Keyless/1  "pwd_Keyless"
  Panel_Lock/3(pwd_Position) -> pwd_Position/1  "pwd_Position"
  Panel_Lock/2(state_KSPassword) -> state_KSPassword/1  "state_KSPassword"
  Panel_Lock/1(state_KSLock) -> state_KSLock/1  "state_KSLock"
  Panel_Lock/5(KSS_Display) -> KSS_Display/1  "KSS_Display"
  ee_User1Password/1 -> Panel_Lock/5(ee_User1Password)
  ee_User2Password/1 -> Panel_Lock/6(ee_User2Password)
  state_KeylessPanel/1 -> Panel_Lock/2(state_KeylessPanel)  "state_KeylessPanel"
  can_Keypad/1 -> Panel_Lock/1(can_Keypad)  "can_Keypad"
  state_MachineLock/1 -> Panel_Lock/7(state_MachineLock)
  state_Engine/1 -> Panel_Lock/8(state_Engine)
  Panel_Lock/6(PWD_NumberSelected) -> PWD_NumberSelected/1  "PWD_NumberSelected"
  flag_InitEERead/1 -> Panel_Lock/9(flag_InitEERead)

== system MX_Gateway/MX_Gtwy_Control/System/Machine_Lock ==
  ddp_MachineLock : Inport
  state_KeylessPanel : Inport  Port=2
  state_System : Inport  Port=3
  state_DeluxeG5 : Inport  Port=4
  flag_InitEERead : Inport  Port=5
  auth_DeluxeG55 : Inport  Port=6
  state_DeluxeG55 : Inport  Port=7
  state_KSLock : Inport  Port=8
  state_CommDeluxeG55 : Inport  Port=9
  Lock_Status : Stateflow chart  TreatAsAtomicUnit=on
  state_MachineLock : Outport
  wires:
  Lock_Status/1(state_MachineLock) -> state_MachineLock/1  "state_MachineLock"
  ddp_MachineLock/1 -> Lock_Status/1(ddp_MachineLock)  "ddp_MachineLock"
  state_KeylessPanel/1 -> Lock_Status/2(state_KeylessPanel)  "state_KeylessPanel"
  state_KSLock/1 -> Lock_Status/3(state_KSLock)  "state_KSLock"
  state_System/1 -> Lock_Status/4(state_System)  "state_System"
  state_DeluxeG5/1 -> Lock_Status/5(state_DeluxeG5)  "state_DeluxeG5"
  flag_InitEERead/1 -> Lock_Status/6(flag_InitEERead)  "flag_InitEERead"
  state_CommDeluxeG55/1 -> Lock_Status/7(state_CommDeluxeG55)
  auth_DeluxeG55/1 -> Lock_Status/8(auth_DeluxeG55)
  state_DeluxeG55/1 -> Lock_Status/9(state_DeluxeG55)

== system MX_Gateway/MX_Gtwy_Control/System/Throttle_Command ==
  cmd_AutoIdle : Inport
  cmd_CANThrottle : Inport  Port=2
  state_Engine : Inport  Port=3
  pos_Throttle : Inport  Port=4
  state_EOT : Inport  Port=5
  flt_EOP : Inport  Port=6
  state_ecuEOP : Inport  Port=7
  Throttle_Command : Stateflow chart  TreatAsAtomicUnit=on
  cmd_Throttle : Outport
  state_TC : Outport  Port=2
  wires:
  cmd_AutoIdle/1 -> Throttle_Command/1(cmd_AutoIdle)
  cmd_CANThrottle/1 -> Throttle_Command/2(cmd_CANThrottle)
  Throttle_Command/1(cmd_Throttle) -> cmd_Throttle/1
  pos_Throttle/1 -> Throttle_Command/3(pos_Throttle)
  state_EOT/1 -> Throttle_Command/4(state_EOT)
  Throttle_Command/2(state_TC) -> state_TC/1
  state_Engine/1 -> Throttle_Command/5(state_Engine)
  state_ecuEOP/1 -> Throttle_Command/6(state_ecuEOP)
  flt_EOP/1 -> Throttle_Command/7(flt_EOP)

== system MX_Gateway/MX_Gtwy_Control/System/mode_Control_Change ==
  mode_LeftControl : Inport
  mode_RightControl : Inport  Port=2
  aux_Flow : Inport  Port=3
  display_request : Inport  Port=4
  dout_GlowPlugs : Inport  Port=5
  Compare To Constant : simulink/Logic and Bit Operations/Compare To Constant  relop===  const=MODE_LEFT_NONE  OutDataTypeStr=boolean
  Compare To Constant1 : simulink/Logic and Bit Operations/Compare To Constant  relop===  const=MODE_RIGHT_NONE  OutDataTypeStr=boolean
  Detect Change : simulink/Logic and Bit Operations/Detect Change  vinit=0  OutDataTypeStr=boolean
  Detect Change1 : simulink/Logic and Bit Operations/Detect Change  vinit=0  OutDataTypeStr=boolean
  Detect Change2 : simulink/Logic and Bit Operations/Detect Change  vinit=0  OutDataTypeStr=boolean
  Detect Decrease : simulink/Logic and Bit Operations/Detect Decrease  vinit=0  OutDataTypeStr=boolean
  Logical Operator : Logic  Operator=OR  Inputs=5  AllPortsSameDT=off  OutDataTypeStr=boolean
  Logical Operator1 : Logic  Operator=NAND  AllPortsSameDT=off  OutDataTypeStr=boolean
  Logical Operator2 : Logic  AllPortsSameDT=off  OutDataTypeStr=boolean
  mode_ControlChange : Outport
  wires:
  mode_RightControl/1 -> Compare To Constant1/1, Detect Change1/1
  Detect Change/1 -> Logical Operator/1
  Detect Change1/1 -> Logical Operator/2
  mode_LeftControl/1 -> Compare To Constant/1, Detect Change/1
  Detect Change2/1 -> Logical Operator/3
  Compare To Constant/1 -> Logical Operator1/1
  aux_Flow/1 -> Detect Change2/1
  Logical Operator1/1 -> Logical Operator2/1
  Compare To Constant1/1 -> Logical Operator1/2
  display_request/1 -> Logical Operator/4
  Logical Operator/1 -> Logical Operator2/2
  Logical Operator2/1 -> mode_ControlChange/1
  Detect Decrease/1 -> Logical Operator/5
  dout_GlowPlugs/1 -> Detect Decrease/1

== system MX_Gateway/MX_Gtwy_Control/Inputs/Aux_Button/AuxButtonLogic ==
  button_Aux_Display : Inport
  can_AuxEnable_ST : Inport  Port=2
  can_AuxEnable_ACD : Inport  Port=3
  state_Buttons : Inport  Port=4
  state_Console : Inport  Port=5
  Aux_Pot : Stateflow chart  TreatAsAtomicUnit=on
  CompareToConstant1 : simulink/Logic and Bit Operations/Compare To Constant  relop=~=  const=NON_FUNCTIONAL  OutDataTypeStr=boolean  ZeroCross=off
  CompareToConstant2 : simulink/Logic and Bit Operations/Compare To Constant  relop=~=  const=NON_FUNCTIONAL  OutDataTypeStr=boolean  ZeroCross=off
  Constant1 : Constant  Value=CD_STAUX_FUNCTION
  Constant2 : Constant  Value=CD_RACD_FUNCTION
  Detect Increase : simulink/Logic and Bit Operations/Detect Increase  vinit=0.0  InputProcessing=Inherited  OutDataTypeStr=uint8
  Detect Increase1 : simulink/Logic and Bit Operations/Detect Increase  vinit=0.0  InputProcessing=Inherited  OutDataTypeStr=uint8
  Logical Operator : Logic  Operator=OR  Inputs=3  AllPortsSameDT=off  OutDataTypeStr=boolean
  Logical Operator1 : Logic  AllPortsSameDT=off  OutDataTypeStr=boolean
  Logical Operator2 : Logic  AllPortsSameDT=off  OutDataTypeStr=boolean
  aux_DebounceButton : Outport
  aux_DetentHold : Outport  Port=2
  wires:
  button_Aux_Display/1 -> Logical Operator/1
  can_AuxEnable_ST/1 -> Detect Increase1/1
  can_AuxEnable_ACD/1 -> Detect Increase/1
  Detect Increase1/1 -> Logical Operator1/1
  Logical Operator1/1 -> Logical Operator/2
  Constant1/1 -> CompareToConstant1/1
  CompareToConstant1/1 -> Logical Operator1/2
  Constant2/1 -> CompareToConstant2/1
  CompareToConstant2/1 -> Logical Operator2/2
  Detect Increase/1 -> Logical Operator2/1
  Logical Operator2/1 -> Logical Operator/3
  Aux_Pot/2(aux_DetentHold) -> aux_DetentHold/1
  state_Buttons/1 -> Aux_Pot/2(state_Buttons)
  state_Console/1 -> Aux_Pot/3(state_Console)
  Aux_Pot/1(aux_DebounceButton) -> aux_DebounceButton/1
  Logical Operator/1 -> Aux_Pot/1(aux_Button)

== system MX_Gateway/MX_Gtwy_Control/Inputs/Aux_Pot/Aux_Pot ==
  state_AuxEnable : Inport
  aux_DebounceButton : Inport  Port=2
  aux_DetentHold : Inport  Port=3
  ain_AuxPot : Inport  Port=4
  ain_SensorSupply2 : Inport  Port=5
  state_Console : Inport  Port=6
  state_Engine : Inport  Port=7
  aux_Flow : Inport  Port=8
  state_SensorSupply2 : Inport  Port=9
  Enable : EnablePort  StatesWhenEnabling=reset
  Aux_Pot : Stateflow chart  TreatAsAtomicUnit=on
  Data Type Conversion : DataTypeConversion  OutDataTypeStr=int16
  Data Type Conversion3 : DataTypeConversion  OutDataTypeStr=uint16
  Pot Ratio : Subsystem
  Saturation : Saturate  UpperLimit=1000  LowerLimit=0
      settings: AttributesFormatString=Upper Limit = %<UpperLimit>\nLower Limit = %<LowerLimit>
  state_AuxPot : Outport  OutputWhenDisabled=reset  InitialOutput=[INIT_NORMAL]
  position_AuxPot : Outport  Port=2  OutputWhenDisabled=reset  InitialOutput=[500]
  rm_AuxPot : Outport  Port=3  OutputWhenDisabled=reset  InitialOutput=[500]
  wires:
  Aux_Pot/1(state_AuxPot) -> state_AuxPot/1
  Aux_Pot/2(position_AuxPotOut) -> Data Type Conversion3/1
  ain_AuxPot/1 -> Pot Ratio/1(ain_AuxPot)
  Data Type Conversion/1 -> Aux_Pot/8(rm_AuxPot)
  Saturation/1 -> position_AuxPot/1
  state_AuxEnable/1 -> Aux_Pot/3(state_AuxEnable)
  Pot Ratio/1(rm_AuxPot) -> Data Type Conversion/1, rm_AuxPot/1
  ain_SensorSupply2/1 -> Pot Ratio/2(ain_SensorSupply2)
  state_Console/1 -> Aux_Pot/5(state_Console)
  state_Engine/1 -> Aux_Pot/6(state_Engine)
  aux_Flow/1 -> Aux_Pot/2(aux_Flow)
  state_SensorSupply2/1 -> Aux_Pot/7(state_SensorSupply)
  Data Type Conversion3/1 -> Saturation/1
  aux_DebounceButton/1 -> Aux_Pot/1(aux_DebounceButton)
  aux_DetentHold/1 -> Aux_Pot/4(aux_DetentHold)

== system MX_Gateway/MX_Gtwy_Control/Inputs/Aux_Pot/CAN_Aux_Pot ==
  state_AuxEnable : Inport
  aux_DebounceButton : Inport  Port=2
  aux_DetentHold : Inport  Port=3
  state_Console : Inport  Port=4
  state_Engine : Inport  Port=5
  aux_Flow : Inport  Port=6
  cmd_PotRight : Inport  Port=7
  msgAge_BJM2Right : Inport  Port=8
  Enable : EnablePort  StatesWhenEnabling=reset
  Aux_Pot : Stateflow chart  TreatAsAtomicUnit=on
  Constant : Constant  Value=500  OutDataTypeStr=int16
  Data Type Conversion : DataTypeConversion  OutDataTypeStr=uint16
  Data Type Conversion3 : DataTypeConversion  OutDataTypeStr=uint16
  Gain : Gain  Gain=1/2  OutDataTypeStr=int16
  Saturation : Saturate  UpperLimit=1000  LowerLimit=0
      settings: AttributesFormatString=Upper Limit = %<UpperLimit>\nLower Limit = %<LowerLimit>
  Sum : Sum  Inputs=|++
  Unary Minus : UnaryMinus
  state_AuxPot : Outport  OutputWhenDisabled=reset  InitialOutput=[INIT_NORMAL]
  position_AuxPot : Outport  Port=2  OutputWhenDisabled=reset  InitialOutput=[500]
  rm_AuxPot : Outport  Port=3  OutputWhenDisabled=reset  InitialOutput=[500]
  wires:
  Aux_Pot/1(state_AuxPot) -> state_AuxPot/1
  Aux_Pot/2(position_AuxPotOut) -> Data Type Conversion3/1
  Saturation/1 -> position_AuxPot/1
  state_AuxEnable/1 -> Aux_Pot/3(state_AuxEnable)
  state_Console/1 -> Aux_Pot/5(state_Console)
  state_Engine/1 -> Aux_Pot/6(state_Engine)
  aux_Flow/1 -> Aux_Pot/2(aux_Flow)
  Data Type Conversion/1 -> rm_AuxPot/1
  cmd_PotRight/1 -> Unary Minus/1
  Constant/1 -> Sum/2
  Sum/1 -> Aux_Pot/8(rm_AuxPot), Data Type Conversion/1
  Gain/1 -> Sum/1
  msgAge_BJM2Right/1 -> Aux_Pot/7(msgAge_BJM2Right)
  Unary Minus/1 -> Gain/1
  Data Type Conversion3/1 -> Saturation/1
  aux_DebounceButton/1 -> Aux_Pot/1(aux_DebounceButton)
  aux_DetentHold/1 -> Aux_Pot/4(aux_DetentHold)

== system MX_Gateway/MX_Gtwy_Control/Inputs/ECT/ConvertToRatio ==
  ain_ECT : Inport  OutDataTypeStr=uint16
  ain_SensorSupply1 : Inport  Port=2  OutDataTypeStr=uint16
  Enable : EnablePort  StatesWhenEnabling=reset  PropagateVarSize=During execution
  Constant : Constant  Value=CD_ECT_FILTER_GAIN  OutDataTypeStr=uint8
  Data Type Conversion1 : DataTypeConversion
  Data Type Conversion2 : DataTypeConversion
  Data Type Conversion3 : DataTypeConversion  OutDataTypeStr=uint16
  GaugeTable : Lookup  InputValues=MXGTW_ECTSNDINPUT  Table=MXGTW_ECTSNDOUTPUT  LookUpMeth=Use Input Nearest  OutDataTypeStr=uint16  RndMeth=Zero  SaturateOnIntegerOverflow=on
      settings: AttributesFormatString=input = %<InputValues>\noutput = %<OutputValues>\nlookup = %<LookUpMeth>\nrounding = %<RndMeth>
  LP_Filter : MX_Library/LP_Filter  GAIN=3
  LP_Filter_Adj_Gain : MX_Library/LP_Filter_Adj_Gain
  Ratiometric_Converter : MX_Library/Ratiometric_Converter  multiplier=1000
  Remove_OOR_Levels : MX_Library/Remove_OOR_Levels  OORL_LEVEL=CD_ECT_OORL  OORH_LEVEL=CD_ECT_OORH
  rm_ECT : Outport  InitialOutput=[0]
  flt_ECT_in : Outport  Port=2  InitialOutput=[0]
  wires:
  ain_ECT/1 -> Ratiometric_Converter/1  "ain_ECT"
  ain_SensorSupply1/1 -> Ratiometric_Converter/2  "ain_SensorSupply1"
  Ratiometric_Converter/1 -> Remove_OOR_Levels/1, LP_Filter/1
  GaugeTable/1 -> flt_ECT_in/1  "flt_ECT_in"
  Data Type Conversion1/1 -> rm_ECT/1  "rm_ECT"
  Remove_OOR_Levels/1 -> Data Type Conversion2/1
  LP_Filter/1 -> Data Type Conversion1/1
  Constant/1 -> LP_Filter_Adj_Gain/1
  Data Type Conversion2/1 -> LP_Filter_Adj_Gain/2
  LP_Filter_Adj_Gain/1 -> Data Type Conversion3/1
  Data Type Conversion3/1 -> GaugeTable/1

== system MX_Gateway/MX_Gtwy_Control/Inputs/EMEA_Left_Control/Normal_Pot ==
  ain_LeftPot : Inport
  ain_SensorSupply2 : Inport  Port=2
  state_Console : Inport  Port=3
  state_Engine : Inport  Port=4
  state_JoystickEnable : Inport  Port=5
  state_SensorSupply2 : Inport  Port=6
  check_LeftNeutral : Inport  Port=7
  Enable : EnablePort  StatesWhenEnabling=reset  PropagateVarSize=During execution
  Data Type Conversion : DataTypeConversion  OutDataTypeStr=int16
  Pot : Stateflow chart  TreatAsAtomicUnit=on
  Pot Ratio : Subsystem
  state_LeftPot : Outport  OutputWhenDisabled=reset  InitialOutput=[INIT_NORMAL]
  position_LeftPot : Outport  Port=2  OutputWhenDisabled=reset  InitialOutput=[500]
  rm_LeftPot : Outport  Port=3  OutputWhenDisabled=reset  InitialOutput=[500]
  wires:
  ain_LeftPot/1 -> Pot Ratio/1(ain_LeftPot)
  Pot/1(state_LeftPot) -> state_LeftPot/1
  ain_SensorSupply2/1 -> Pot Ratio/2(ain_SensorSupply2)
  state_Console/1 -> Pot/4(state_Console)
  Pot/2(position_LeftPotOut) -> position_LeftPot/1
  state_Engine/1 -> Pot/5(state_Engine)
  Data Type Conversion/1 -> Pot/1(rm_LeftPot)
  state_JoystickEnable/1 -> Pot/6(state_JoystickEnable)
  state_SensorSupply2/1 -> Pot/3(state_SensorSupply)
  check_LeftNeutral/1 -> Pot/7(check_LeftNeutral)
  Pot Ratio/1(rm_LeftPot) -> rm_LeftPot/1, Data Type Conversion/1
  Pot Ratio/2(raw_LeftPot) -> Pot/2(raw_LeftPot)

== system MX_Gateway/MX_Gtwy_Control/Inputs/EMEA_Right_Control/Right Pot ==
  state_AuxEnable : Inport
  aux_Flow : Inport  Port=2
  state_Engine : Inport  Port=3
  ain_RightPot : Inport  Port=4
  ain_SensorSupply2 : Inport  Port=5
  aux_DetentHold : Inport  Port=6
  aux_DebounceButton : Inport  Port=7
  state_Console : Inport  Port=8
  state_SensorSupply2 : Inport  Port=9
  check_RightNeutral : Inport  Port=10
  aux_Inhibit : Inport  Port=11
  mode_RightControl : Inport  Port=12
  Enable : EnablePort  StatesWhenEnabling=reset
  Data Type Conversion : DataTypeConversion  OutDataTypeStr=int16
  Data Type Conversion3 : DataTypeConversion  OutDataTypeStr=uint16
  Pot Ratio : Subsystem
  Right_Pot : Stateflow chart  TreatAsAtomicUnit=on
  Saturation : Saturate  UpperLimit=1000  LowerLimit=0
      settings: AttributesFormatString=Upper Limit = %<UpperLimit>\nLower Limit = %<LowerLimit>
  state_RightPot : Outport  OutputWhenDisabled=reset  InitialOutput=[INIT_NORMAL]
  position_RightPot : Outport  Port=2  OutputWhenDisabled=reset  InitialOutput=[500]
  rm_RightPot : Outport  Port=3  OutputWhenDisabled=reset  InitialOutput=[500]
  wires:
  Right_Pot/1(state_RightPot) -> state_RightPot/1
  Right_Pot/2(position_RightPotOut) -> Data Type Conversion3/1
  ain_RightPot/1 -> Pot Ratio/1(ain_RightPot)
  Data Type Conversion/1 -> Right_Pot/12(rm_RightPot)
  Saturation/1 -> position_RightPot/1
  state_AuxEnable/1 -> Right_Pot/3(state_AuxEnable)
  Pot Ratio/2(rm_RightPot) -> Data Type Conversion/1, rm_RightPot/1
  ain_SensorSupply2/1 -> Pot Ratio/2(ain_SensorSupply2)
  state_Console/1 -> Right_Pot/5(state_Console)
  state_Engine/1 -> Right_Pot/6(state_Engine)
  aux_Flow/1 -> Right_Pot/2(aux_Flow)
  state_SensorSupply2/1 -> Right_Pot/7(state_SensorSupply)
  Data Type Conversion3/1 -> Saturation/1
  aux_DebounceButton/1 -> Right_Pot/1(aux_DebounceButton)
  aux_DetentHold/1 -> Right_Pot/4(aux_DetentHold)
  check_RightNeutral/1 -> Right_Pot/9(check_RightNeutral)
  aux_Inhibit/1 -> Right_Pot/8(aux_Inhibit)
  mode_RightControl/1 -> Right_Pot/10(mode_RightControl)
  Pot Ratio/1(raw_RightPot) -> Right_Pot/11(raw_RightPot)
